simulink library "Garbage"  (R2023a)
settings: Solver=ode45  StartTime=0.0  StopTime=10.0  RelTol=1e-3  EnableMultiTasking=on
undefined symbols (not defined in the file or in its folder): c, ending_column_index, ending_row_index, framebuffer_16x1, framebuffer_2x2, framebuffer_4x4, framebuffer_8x8, HEIGHT, hot, POS_X, POS_Y, starting_column_index, starting_row_index, WIDTH
libraries: Memory_Blocks_Lib x459, simulink x235, simulink_extras x132, dspmtrx3 x116, Bit_Multiplexers_Lib x67, Bit_Demultiplexers_Lib x49, Byte_Multiplexers_Lib x40, Miscellaneous x38, Computer_Library x17, Decoders_Lib x14, commutil2 x12, Arithmetic_Operations_Lib x8
(part 17 of 26)

== system Garbage/Selector_01 ==
  Addr(1B) : Inport
  Bus Selector : BusSelector  OutputSignals=signal1,signal2,signal3,signal4,signal5,signal6,signal7,signal8
  Logical Operator{1..6,26} (7 alike) : Logic  Operator=NOT  Inputs=1  IconShape=distinctive  AllPortsSameDT=off  OutDataTypeStr=boolean
  Logical Operator9 : Logic  Inputs=8  IconShape=distinctive  AllPortsSameDT=off  OutDataTypeStr=boolean
  Select_1(1b) : Outport
  wires:
  Bus Selector/8 -> Logical Operator9/8  "<signal8>"
  Bus Selector/7 -> Logical Operator6/1  "<signal7>"
  Logical Operator6/1 -> Logical Operator9/7
  Bus Selector/6 -> Logical Operator5/1  "<signal6>"
  Logical Operator5/1 -> Logical Operator9/6
  Bus Selector/5 -> Logical Operator4/1  "<signal5>"
  Logical Operator4/1 -> Logical Operator9/5
  Bus Selector/4 -> Logical Operator3/1  "<signal4>"
  Logical Operator3/1 -> Logical Operator9/4
  Bus Selector/3 -> Logical Operator2/1  "<signal3>"
  Logical Operator2/1 -> Logical Operator9/3
  Bus Selector/2 -> Logical Operator1/1  "<signal2>"
  Logical Operator1/1 -> Logical Operator9/2
  Logical Operator9/1 -> Select_1(1b)/1
  Bus Selector/1 -> Logical Operator26/1  "<signal1>"
  Logical Operator26/1 -> Logical Operator9/1
  Addr(1B)/1 -> Bus Selector/1

== system Garbage/Selector_02 ==
  Addr(1B) : Inport
  Bus Selector : BusSelector  OutputSignals=signal1,signal2,signal3,signal4,signal5,signal6,signal7,signal8
  Logical Operator{1..5,7,26} (7 alike) : Logic  Operator=NOT  Inputs=1  IconShape=distinctive  AllPortsSameDT=off  OutDataTypeStr=boolean
  Logical Operator9 : Logic  Inputs=8  IconShape=distinctive  AllPortsSameDT=off  OutDataTypeStr=boolean
  Select_2(1b) : Outport
  wires:
  Bus Selector/8 -> Logical Operator7/1  "<signal8>"
  Logical Operator7/1 -> Logical Operator9/8
  Bus Selector/7 -> Logical Operator9/7  "<signal7>"
  Bus Selector/6 -> Logical Operator5/1  "<signal6>"
  Logical Operator5/1 -> Logical Operator9/6
  Bus Selector/5 -> Logical Operator4/1  "<signal5>"
  Logical Operator4/1 -> Logical Operator9/5
  Bus Selector/4 -> Logical Operator3/1  "<signal4>"
  Logical Operator3/1 -> Logical Operator9/4
  Bus Selector/3 -> Logical Operator2/1  "<signal3>"
  Logical Operator2/1 -> Logical Operator9/3
  Bus Selector/2 -> Logical Operator1/1  "<signal2>"
  Logical Operator1/1 -> Logical Operator9/2
  Logical Operator9/1 -> Select_2(1b)/1
  Bus Selector/1 -> Logical Operator26/1  "<signal1>"
  Logical Operator26/1 -> Logical Operator9/1
  Addr(1B)/1 -> Bus Selector/1

== system Garbage/Selector_03 ==
  Addr(1B) : Inport
  Bus Selector : BusSelector  OutputSignals=signal1,signal2,signal3,signal4,signal5,signal6,signal7,signal8
  Logical Operator{1..5,26} (6 alike) : Logic  Operator=NOT  Inputs=1  IconShape=distinctive  AllPortsSameDT=off  OutDataTypeStr=boolean
  Logical Operator9 : Logic  Inputs=8  IconShape=distinctive  AllPortsSameDT=off  OutDataTypeStr=boolean
  Select_3(1b) : Outport
  wires:
  Bus Selector/8 -> Logical Operator9/8  "<signal8>"
  Bus Selector/7 -> Logical Operator9/7  "<signal7>"
  Bus Selector/6 -> Logical Operator5/1  "<signal6>"
  Logical Operator5/1 -> Logical Operator9/6
  Bus Selector/5 -> Logical Operator4/1  "<signal5>"
  Logical Operator4/1 -> Logical Operator9/5
  Bus Selector/4 -> Logical Operator3/1  "<signal4>"
  Logical Operator3/1 -> Logical Operator9/4
  Bus Selector/3 -> Logical Operator2/1  "<signal3>"
  Logical Operator2/1 -> Logical Operator9/3
  Bus Selector/2 -> Logical Operator1/1  "<signal2>"
  Logical Operator1/1 -> Logical Operator9/2
  Logical Operator9/1 -> Select_3(1b)/1
  Bus Selector/1 -> Logical Operator26/1  "<signal1>"
  Logical Operator26/1 -> Logical Operator9/1
  Addr(1B)/1 -> Bus Selector/1

== system Garbage/Selector_04 ==
  Addr(1B) : Inport
  Bus Selector : BusSelector  OutputSignals=signal1,signal2,signal3,signal4,signal5,signal6,signal7,signal8
  Logical Operator{1..4,6..7,26} (7 alike) : Logic  Operator=NOT  Inputs=1  IconShape=distinctive  AllPortsSameDT=off  OutDataTypeStr=boolean
  Logical Operator9 : Logic  Inputs=8  IconShape=distinctive  AllPortsSameDT=off  OutDataTypeStr=boolean
  Select_4(1b) : Outport
  wires:
  Bus Selector/8 -> Logical Operator7/1  "<signal8>"
  Logical Operator7/1 -> Logical Operator9/8
  Bus Selector/7 -> Logical Operator6/1  "<signal7>"
  Logical Operator6/1 -> Logical Operator9/7
  Bus Selector/6 -> Logical Operator9/6  "<signal6>"
  Bus Selector/5 -> Logical Operator4/1  "<signal5>"
  Logical Operator4/1 -> Logical Operator9/5
  Bus Selector/4 -> Logical Operator3/1  "<signal4>"
  Logical Operator3/1 -> Logical Operator9/4
  Bus Selector/3 -> Logical Operator2/1  "<signal3>"
  Logical Operator2/1 -> Logical Operator9/3
  Bus Selector/2 -> Logical Operator1/1  "<signal2>"
  Logical Operator1/1 -> Logical Operator9/2
  Logical Operator9/1 -> Select_4(1b)/1
  Bus Selector/1 -> Logical Operator26/1  "<signal1>"
  Logical Operator26/1 -> Logical Operator9/1
  Addr(1B)/1 -> Bus Selector/1

== system Garbage/Selector_05 ==
  Addr(1B) : Inport
  Bus Selector : BusSelector  OutputSignals=signal1,signal2,signal3,signal4,signal5,signal6,signal7,signal8
  Logical Operator{1..4,6,26} (6 alike) : Logic  Operator=NOT  Inputs=1  IconShape=distinctive  AllPortsSameDT=off  OutDataTypeStr=boolean
  Logical Operator9 : Logic  Inputs=8  IconShape=distinctive  AllPortsSameDT=off  OutDataTypeStr=boolean
  Select_5(1b) : Outport
  wires:
  Bus Selector/8 -> Logical Operator9/8  "<signal8>"
  Bus Selector/7 -> Logical Operator6/1  "<signal7>"
  Logical Operator6/1 -> Logical Operator9/7
  Bus Selector/6 -> Logical Operator9/6  "<signal6>"
  Bus Selector/5 -> Logical Operator4/1  "<signal5>"
  Logical Operator4/1 -> Logical Operator9/5
  Bus Selector/4 -> Logical Operator3/1  "<signal4>"
  Logical Operator3/1 -> Logical Operator9/4
  Bus Selector/3 -> Logical Operator2/1  "<signal3>"
  Logical Operator2/1 -> Logical Operator9/3
  Bus Selector/2 -> Logical Operator1/1  "<signal2>"
  Logical Operator1/1 -> Logical Operator9/2
  Logical Operator9/1 -> Select_5(1b)/1
  Bus Selector/1 -> Logical Operator26/1  "<signal1>"
  Logical Operator26/1 -> Logical Operator9/1
  Addr(1B)/1 -> Bus Selector/1

== system Garbage/Selector_06 ==
  Addr(1B) : Inport
  Bus Selector : BusSelector  OutputSignals=signal1,signal2,signal3,signal4,signal5,signal6,signal7,signal8
  Logical Operator{1..4,7,26} (6 alike) : Logic  Operator=NOT  Inputs=1  IconShape=distinctive  AllPortsSameDT=off  OutDataTypeStr=boolean
  Logical Operator9 : Logic  Inputs=8  IconShape=distinctive  AllPortsSameDT=off  OutDataTypeStr=boolean
  Select_6(1b) : Outport
  wires:
  Bus Selector/8 -> Logical Operator7/1  "<signal8>"
  Logical Operator7/1 -> Logical Operator9/8
  Bus Selector/7 -> Logical Operator9/7  "<signal7>"
  Bus Selector/6 -> Logical Operator9/6  "<signal6>"
  Bus Selector/5 -> Logical Operator4/1  "<signal5>"
  Logical Operator4/1 -> Logical Operator9/5
  Bus Selector/4 -> Logical Operator3/1  "<signal4>"
  Logical Operator3/1 -> Logical Operator9/4
  Bus Selector/3 -> Logical Operator2/1  "<signal3>"
  Logical Operator2/1 -> Logical Operator9/3
  Bus Selector/2 -> Logical Operator1/1  "<signal2>"
  Logical Operator1/1 -> Logical Operator9/2
  Logical Operator9/1 -> Select_6(1b)/1
  Bus Selector/1 -> Logical Operator26/1  "<signal1>"
  Logical Operator26/1 -> Logical Operator9/1
  Addr(1B)/1 -> Bus Selector/1

== system Garbage/Selector_07 ==
  Addr(1B) : Inport
  Bus Selector : BusSelector  OutputSignals=signal1,signal2,signal3,signal4,signal5,signal6,signal7,signal8
  Logical Operator{1..4,26} (5 alike) : Logic  Operator=NOT  Inputs=1  IconShape=distinctive  AllPortsSameDT=off  OutDataTypeStr=boolean
  Logical Operator9 : Logic  Inputs=8  IconShape=distinctive  AllPortsSameDT=off  OutDataTypeStr=boolean
  Select_7(1b) : Outport
  wires:
  Bus Selector/8 -> Logical Operator9/8  "<signal8>"
  Bus Selector/7 -> Logical Operator9/7  "<signal7>"
  Bus Selector/6 -> Logical Operator9/6  "<signal6>"
  Bus Selector/5 -> Logical Operator4/1  "<signal5>"
  Logical Operator4/1 -> Logical Operator9/5
  Bus Selector/4 -> Logical Operator3/1  "<signal4>"
  Logical Operator3/1 -> Logical Operator9/4
  Bus Selector/3 -> Logical Operator2/1  "<signal3>"
  Logical Operator2/1 -> Logical Operator9/3
  Bus Selector/2 -> Logical Operator1/1  "<signal2>"
  Logical Operator1/1 -> Logical Operator9/2
  Logical Operator9/1 -> Select_7(1b)/1
  Bus Selector/1 -> Logical Operator26/1  "<signal1>"
  Logical Operator26/1 -> Logical Operator9/1
  Addr(1B)/1 -> Bus Selector/1

== system Garbage/Selector_08 ==
  Addr(1B) : Inport
  Bus Selector : BusSelector  OutputSignals=signal1,signal2,signal3,signal4,signal5,signal6,signal7,signal8
  Logical Operator{1..3,5..7,26} (7 alike) : Logic  Operator=NOT  Inputs=1  IconShape=distinctive  AllPortsSameDT=off  OutDataTypeStr=boolean
  Logical Operator9 : Logic  Inputs=8  IconShape=distinctive  AllPortsSameDT=off  OutDataTypeStr=boolean
  Select_8(1b) : Outport
  wires:
  Bus Selector/8 -> Logical Operator7/1  "<signal8>"
  Logical Operator7/1 -> Logical Operator9/8
  Bus Selector/7 -> Logical Operator6/1  "<signal7>"
  Logical Operator6/1 -> Logical Operator9/7
  Bus Selector/6 -> Logical Operator5/1  "<signal6>"
  Logical Operator5/1 -> Logical Operator9/6
  Bus Selector/5 -> Logical Operator9/5  "<signal5>"
  Bus Selector/4 -> Logical Operator3/1  "<signal4>"
  Logical Operator3/1 -> Logical Operator9/4
  Bus Selector/3 -> Logical Operator2/1  "<signal3>"
  Logical Operator2/1 -> Logical Operator9/3
  Bus Selector/2 -> Logical Operator1/1  "<signal2>"
  Logical Operator1/1 -> Logical Operator9/2
  Logical Operator9/1 -> Select_8(1b)/1
  Bus Selector/1 -> Logical Operator26/1  "<signal1>"
  Logical Operator26/1 -> Logical Operator9/1
  Addr(1B)/1 -> Bus Selector/1

== system Garbage/Selector_09 ==
  Addr(1B) : Inport
  Bus Selector : BusSelector  OutputSignals=signal1,signal2,signal3,signal4,signal5,signal6,signal7,signal8
  Logical Operator{1..3,5..6,26} (6 alike) : Logic  Operator=NOT  Inputs=1  IconShape=distinctive  AllPortsSameDT=off  OutDataTypeStr=boolean
  Logical Operator9 : Logic  Inputs=8  IconShape=distinctive  AllPortsSameDT=off  OutDataTypeStr=boolean
  Select_9(1b) : Outport
  wires:
  Bus Selector/8 -> Logical Operator9/8  "<signal8>"
  Bus Selector/7 -> Logical Operator6/1  "<signal7>"
  Logical Operator6/1 -> Logical Operator9/7
  Bus Selector/6 -> Logical Operator5/1  "<signal6>"
  Logical Operator5/1 -> Logical Operator9/6
  Bus Selector/5 -> Logical Operator9/5  "<signal5>"
  Bus Selector/4 -> Logical Operator3/1  "<signal4>"
  Logical Operator3/1 -> Logical Operator9/4
  Bus Selector/3 -> Logical Operator2/1  "<signal3>"
  Logical Operator2/1 -> Logical Operator9/3
  Bus Selector/2 -> Logical Operator1/1  "<signal2>"
  Logical Operator1/1 -> Logical Operator9/2
  Logical Operator9/1 -> Select_9(1b)/1
  Bus Selector/1 -> Logical Operator26/1  "<signal1>"
  Logical Operator26/1 -> Logical Operator9/1
  Addr(1B)/1 -> Bus Selector/1

== system Garbage/Selector_10 ==
  Addr(1B) : Inport
  Bus Selector : BusSelector  OutputSignals=signal1,signal2,signal3,signal4,signal5,signal6,signal7,signal8
  Logical Operator{1..3,5,7,26} (6 alike) : Logic  Operator=NOT  Inputs=1  IconShape=distinctive  AllPortsSameDT=off  OutDataTypeStr=boolean
  Logical Operator9 : Logic  Inputs=8  IconShape=distinctive  AllPortsSameDT=off  OutDataTypeStr=boolean
  Select_10(1b) : Outport
  wires:
  Bus Selector/8 -> Logical Operator7/1  "<signal8>"
  Logical Operator7/1 -> Logical Operator9/8
  Bus Selector/7 -> Logical Operator9/7  "<signal7>"
  Bus Selector/6 -> Logical Operator5/1  "<signal6>"
  Logical Operator5/1 -> Logical Operator9/6
  Bus Selector/5 -> Logical Operator9/5  "<signal5>"
  Bus Selector/4 -> Logical Operator3/1  "<signal4>"
  Logical Operator3/1 -> Logical Operator9/4
  Bus Selector/3 -> Logical Operator2/1  "<signal3>"
  Logical Operator2/1 -> Logical Operator9/3
  Bus Selector/2 -> Logical Operator1/1  "<signal2>"
  Logical Operator1/1 -> Logical Operator9/2
  Logical Operator9/1 -> Select_10(1b)/1
  Bus Selector/1 -> Logical Operator26/1  "<signal1>"
  Logical Operator26/1 -> Logical Operator9/1
  Addr(1B)/1 -> Bus Selector/1

== system Garbage/Selector_11 ==
  Addr(1B) : Inport
  Bus Selector : BusSelector  OutputSignals=signal1,signal2,signal3,signal4,signal5,signal6,signal7,signal8
  Logical Operator{1..3,5,26} (5 alike) : Logic  Operator=NOT  Inputs=1  IconShape=distinctive  AllPortsSameDT=off  OutDataTypeStr=boolean
  Logical Operator9 : Logic  Inputs=8  IconShape=distinctive  AllPortsSameDT=off  OutDataTypeStr=boolean
  Select_11(1b) : Outport
  wires:
  Bus Selector/8 -> Logical Operator9/8  "<signal8>"
  Bus Selector/7 -> Logical Operator9/7  "<signal7>"
  Bus Selector/6 -> Logical Operator5/1  "<signal6>"
  Logical Operator5/1 -> Logical Operator9/6
  Bus Selector/5 -> Logical Operator9/5  "<signal5>"
  Bus Selector/4 -> Logical Operator3/1  "<signal4>"
  Logical Operator3/1 -> Logical Operator9/4
  Bus Selector/3 -> Logical Operator2/1  "<signal3>"
  Logical Operator2/1 -> Logical Operator9/3
  Bus Selector/2 -> Logical Operator1/1  "<signal2>"
  Logical Operator1/1 -> Logical Operator9/2
  Logical Operator9/1 -> Select_11(1b)/1
  Bus Selector/1 -> Logical Operator26/1  "<signal1>"
  Logical Operator26/1 -> Logical Operator9/1
  Addr(1B)/1 -> Bus Selector/1

== system Garbage/Selector_12 ==
  Addr(1B) : Inport
  Bus Selector : BusSelector  OutputSignals=signal1,signal2,signal3,signal4,signal5,signal6,signal7,signal8
  Logical Operator{1..3,6..7,26} (6 alike) : Logic  Operator=NOT  Inputs=1  IconShape=distinctive  AllPortsSameDT=off  OutDataTypeStr=boolean
  Logical Operator9 : Logic  Inputs=8  IconShape=distinctive  AllPortsSameDT=off  OutDataTypeStr=boolean
  Select_12(1b) : Outport
  wires:
  Bus Selector/8 -> Logical Operator7/1  "<signal8>"
  Logical Operator7/1 -> Logical Operator9/8
  Bus Selector/7 -> Logical Operator6/1  "<signal7>"
  Logical Operator6/1 -> Logical Operator9/7
  Bus Selector/6 -> Logical Operator9/6  "<signal6>"
  Bus Selector/5 -> Logical Operator9/5  "<signal5>"
  Bus Selector/4 -> Logical Operator3/1  "<signal4>"
  Logical Operator3/1 -> Logical Operator9/4
  Bus Selector/3 -> Logical Operator2/1  "<signal3>"
  Logical Operator2/1 -> Logical Operator9/3
  Bus Selector/2 -> Logical Operator1/1  "<signal2>"
  Logical Operator1/1 -> Logical Operator9/2
  Logical Operator9/1 -> Select_12(1b)/1
  Bus Selector/1 -> Logical Operator26/1  "<signal1>"
  Logical Operator26/1 -> Logical Operator9/1
  Addr(1B)/1 -> Bus Selector/1

== system Garbage/Selector_13 ==
  Addr(1B) : Inport
  Bus Selector : BusSelector  OutputSignals=signal1,signal2,signal3,signal4,signal5,signal6,signal7,signal8
  Logical Operator{1..3,6,26} (5 alike) : Logic  Operator=NOT  Inputs=1  IconShape=distinctive  AllPortsSameDT=off  OutDataTypeStr=boolean
  Logical Operator9 : Logic  Inputs=8  IconShape=distinctive  AllPortsSameDT=off  OutDataTypeStr=boolean
  Select_13(1b) : Outport
  wires:
  Bus Selector/8 -> Logical Operator9/8  "<signal8>"
  Bus Selector/7 -> Logical Operator6/1  "<signal7>"
  Logical Operator6/1 -> Logical Operator9/7
  Bus Selector/6 -> Logical Operator9/6  "<signal6>"
  Bus Selector/5 -> Logical Operator9/5  "<signal5>"
  Bus Selector/4 -> Logical Operator3/1  "<signal4>"
  Logical Operator3/1 -> Logical Operator9/4
  Bus Selector/3 -> Logical Operator2/1  "<signal3>"
  Logical Operator2/1 -> Logical Operator9/3
  Bus Selector/2 -> Logical Operator1/1  "<signal2>"
  Logical Operator1/1 -> Logical Operator9/2
  Logical Operator9/1 -> Select_13(1b)/1
  Bus Selector/1 -> Logical Operator26/1  "<signal1>"
  Logical Operator26/1 -> Logical Operator9/1
  Addr(1B)/1 -> Bus Selector/1

== system Garbage/Selector_14 ==
  Addr(1B) : Inport
  Bus Selector : BusSelector  OutputSignals=signal1,signal2,signal3,signal4,signal5,signal6,signal7,signal8
  Logical Operator{1..3,7,26} (5 alike) : Logic  Operator=NOT  Inputs=1  IconShape=distinctive  AllPortsSameDT=off  OutDataTypeStr=boolean
  Logical Operator9 : Logic  Inputs=8  IconShape=distinctive  AllPortsSameDT=off  OutDataTypeStr=boolean
  Select_14(1b) : Outport
  wires:
  Bus Selector/8 -> Logical Operator7/1  "<signal8>"
  Logical Operator7/1 -> Logical Operator9/8
  Bus Selector/7 -> Logical Operator9/7  "<signal7>"
  Bus Selector/6 -> Logical Operator9/6  "<signal6>"
  Bus Selector/5 -> Logical Operator9/5  "<signal5>"
  Bus Selector/4 -> Logical Operator3/1  "<signal4>"
  Logical Operator3/1 -> Logical Operator9/4
  Bus Selector/3 -> Logical Operator2/1  "<signal3>"
  Logical Operator2/1 -> Logical Operator9/3
  Bus Selector/2 -> Logical Operator1/1  "<signal2>"
  Logical Operator1/1 -> Logical Operator9/2
  Logical Operator9/1 -> Select_14(1b)/1
  Bus Selector/1 -> Logical Operator26/1  "<signal1>"
  Logical Operator26/1 -> Logical Operator9/1
  Addr(1B)/1 -> Bus Selector/1

== system Garbage/Selector_15 ==
  Addr(1B) : Inport
  Bus Selector : BusSelector  OutputSignals=signal1,signal2,signal3,signal4,signal5,signal6,signal7,signal8
  Logical Operator{1..3,26} (4 alike) : Logic  Operator=NOT  Inputs=1  IconShape=distinctive  AllPortsSameDT=off  OutDataTypeStr=boolean
  Logical Operator9 : Logic  Inputs=8  IconShape=distinctive  AllPortsSameDT=off  OutDataTypeStr=boolean
  Select_15(1b) : Outport
  wires:
  Bus Selector/8 -> Logical Operator9/8  "<signal8>"
  Bus Selector/7 -> Logical Operator9/7  "<signal7>"
  Bus Selector/6 -> Logical Operator9/6  "<signal6>"
  Bus Selector/5 -> Logical Operator9/5  "<signal5>"
  Bus Selector/4 -> Logical Operator3/1  "<signal4>"
  Logical Operator3/1 -> Logical Operator9/4
  Bus Selector/3 -> Logical Operator2/1  "<signal3>"
  Logical Operator2/1 -> Logical Operator9/3
  Bus Selector/2 -> Logical Operator1/1  "<signal2>"
  Logical Operator1/1 -> Logical Operator9/2
  Logical Operator9/1 -> Select_15(1b)/1
  Bus Selector/1 -> Logical Operator26/1  "<signal1>"
  Logical Operator26/1 -> Logical Operator9/1
  Addr(1B)/1 -> Bus Selector/1

== system Garbage/Selector_16 ==
  Addr(1B) : Inport
  Bus Selector : BusSelector  OutputSignals=signal1,signal2,signal3,signal4,signal5,signal6,signal7,signal8
  Logical Operator{1..2,4..7,26} (7 alike) : Logic  Operator=NOT  Inputs=1  IconShape=distinctive  AllPortsSameDT=off  OutDataTypeStr=boolean
  Logical Operator9 : Logic  Inputs=8  IconShape=distinctive  AllPortsSameDT=off  OutDataTypeStr=boolean
  Select_16(1b) : Outport
  wires:
  Bus Selector/8 -> Logical Operator7/1  "<signal8>"
  Logical Operator7/1 -> Logical Operator9/8
  Bus Selector/7 -> Logical Operator6/1  "<signal7>"
  Logical Operator6/1 -> Logical Operator9/7
  Bus Selector/6 -> Logical Operator5/1  "<signal6>"
  Logical Operator5/1 -> Logical Operator9/6
  Bus Selector/5 -> Logical Operator4/1  "<signal5>"
  Logical Operator4/1 -> Logical Operator9/5
  Bus Selector/4 -> Logical Operator9/4  "<signal4>"
  Bus Selector/3 -> Logical Operator2/1  "<signal3>"
  Logical Operator2/1 -> Logical Operator9/3
  Bus Selector/2 -> Logical Operator1/1  "<signal2>"
  Logical Operator1/1 -> Logical Operator9/2
  Logical Operator9/1 -> Select_16(1b)/1
  Bus Selector/1 -> Logical Operator26/1  "<signal1>"
  Logical Operator26/1 -> Logical Operator9/1
  Addr(1B)/1 -> Bus Selector/1

== system Garbage/Selector_17 ==
  Addr(1B) : Inport
  Bus Selector : BusSelector  OutputSignals=signal1,signal2,signal3,signal4,signal5,signal6,signal7,signal8
  Logical Operator{1..2,4..6,26} (6 alike) : Logic  Operator=NOT  Inputs=1  IconShape=distinctive  AllPortsSameDT=off  OutDataTypeStr=boolean
  Logical Operator9 : Logic  Inputs=8  IconShape=distinctive  AllPortsSameDT=off  OutDataTypeStr=boolean
  Select_17(1b) : Outport
  wires:
  Bus Selector/8 -> Logical Operator9/8  "<signal8>"
  Bus Selector/7 -> Logical Operator6/1  "<signal7>"
  Logical Operator6/1 -> Logical Operator9/7
  Bus Selector/6 -> Logical Operator5/1  "<signal6>"
  Logical Operator5/1 -> Logical Operator9/6
  Bus Selector/5 -> Logical Operator4/1  "<signal5>"
  Logical Operator4/1 -> Logical Operator9/5
  Bus Selector/4 -> Logical Operator9/4  "<signal4>"
  Bus Selector/3 -> Logical Operator2/1  "<signal3>"
  Logical Operator2/1 -> Logical Operator9/3
  Bus Selector/2 -> Logical Operator1/1  "<signal2>"
  Logical Operator1/1 -> Logical Operator9/2
  Logical Operator9/1 -> Select_17(1b)/1
  Bus Selector/1 -> Logical Operator26/1  "<signal1>"
  Logical Operator26/1 -> Logical Operator9/1
  Addr(1B)/1 -> Bus Selector/1

== system Garbage/Selector_18 ==
  Addr(1B) : Inport
  Bus Selector : BusSelector  OutputSignals=signal1,signal2,signal3,signal4,signal5,signal6,signal7,signal8
  Logical Operator{1..2,4..5,7,26} (6 alike) : Logic  Operator=NOT  Inputs=1  IconShape=distinctive  AllPortsSameDT=off  OutDataTypeStr=boolean
  Logical Operator9 : Logic  Inputs=8  IconShape=distinctive  AllPortsSameDT=off  OutDataTypeStr=boolean
  Select_18(1b) : Outport
  wires:
  Bus Selector/8 -> Logical Operator7/1  "<signal8>"
  Logical Operator7/1 -> Logical Operator9/8
  Bus Selector/7 -> Logical Operator9/7  "<signal7>"
  Bus Selector/6 -> Logical Operator5/1  "<signal6>"
  Logical Operator5/1 -> Logical Operator9/6
  Bus Selector/5 -> Logical Operator4/1  "<signal5>"
  Logical Operator4/1 -> Logical Operator9/5
  Bus Selector/4 -> Logical Operator9/4  "<signal4>"
  Bus Selector/3 -> Logical Operator2/1  "<signal3>"
  Logical Operator2/1 -> Logical Operator9/3
  Bus Selector/2 -> Logical Operator1/1  "<signal2>"
  Logical Operator1/1 -> Logical Operator9/2
  Logical Operator9/1 -> Select_18(1b)/1
  Bus Selector/1 -> Logical Operator26/1  "<signal1>"
  Logical Operator26/1 -> Logical Operator9/1
  Addr(1B)/1 -> Bus Selector/1

== system Garbage/Selector_19 ==
  Addr(1B) : Inport
  Bus Selector : BusSelector  OutputSignals=signal1,signal2,signal3,signal4,signal5,signal6,signal7,signal8
  Logical Operator{1..2,4..5,26} (5 alike) : Logic  Operator=NOT  Inputs=1  IconShape=distinctive  AllPortsSameDT=off  OutDataTypeStr=boolean
  Logical Operator9 : Logic  Inputs=8  IconShape=distinctive  AllPortsSameDT=off  OutDataTypeStr=boolean
  Select_19(1b) : Outport
  wires:
  Bus Selector/8 -> Logical Operator9/8  "<signal8>"
  Bus Selector/7 -> Logical Operator9/7  "<signal7>"
  Bus Selector/6 -> Logical Operator5/1  "<signal6>"
  Logical Operator5/1 -> Logical Operator9/6
  Bus Selector/5 -> Logical Operator4/1  "<signal5>"
  Logical Operator4/1 -> Logical Operator9/5
  Bus Selector/4 -> Logical Operator9/4  "<signal4>"
  Bus Selector/3 -> Logical Operator2/1  "<signal3>"
  Logical Operator2/1 -> Logical Operator9/3
  Bus Selector/2 -> Logical Operator1/1  "<signal2>"
  Logical Operator1/1 -> Logical Operator9/2
  Logical Operator9/1 -> Select_19(1b)/1
  Bus Selector/1 -> Logical Operator26/1  "<signal1>"
  Logical Operator26/1 -> Logical Operator9/1
  Addr(1B)/1 -> Bus Selector/1

== system Garbage/Selector_20 ==
  Addr(1B) : Inport
  Bus Selector : BusSelector  OutputSignals=signal1,signal2,signal3,signal4,signal5,signal6,signal7,signal8
  Logical Operator{1..2,4,6..7,26} (6 alike) : Logic  Operator=NOT  Inputs=1  IconShape=distinctive  AllPortsSameDT=off  OutDataTypeStr=boolean
  Logical Operator9 : Logic  Inputs=8  IconShape=distinctive  AllPortsSameDT=off  OutDataTypeStr=boolean
  Select_20(1b) : Outport
  wires:
  Bus Selector/8 -> Logical Operator7/1  "<signal8>"
  Logical Operator7/1 -> Logical Operator9/8
  Bus Selector/7 -> Logical Operator6/1  "<signal7>"
  Logical Operator6/1 -> Logical Operator9/7
  Bus Selector/6 -> Logical Operator9/6  "<signal6>"
  Bus Selector/5 -> Logical Operator4/1  "<signal5>"
  Logical Operator4/1 -> Logical Operator9/5
  Bus Selector/4 -> Logical Operator9/4  "<signal4>"
  Bus Selector/3 -> Logical Operator2/1  "<signal3>"
  Logical Operator2/1 -> Logical Operator9/3
  Bus Selector/2 -> Logical Operator1/1  "<signal2>"
  Logical Operator1/1 -> Logical Operator9/2
  Logical Operator9/1 -> Select_20(1b)/1
  Bus Selector/1 -> Logical Operator26/1  "<signal1>"
  Logical Operator26/1 -> Logical Operator9/1
  Addr(1B)/1 -> Bus Selector/1

== system Garbage/Selector_21 ==
  Addr(1B) : Inport
  Bus Selector : BusSelector  OutputSignals=signal1,signal2,signal3,signal4,signal5,signal6,signal7,signal8
  Logical Operator{1..2,4,6,26} (5 alike) : Logic  Operator=NOT  Inputs=1  IconShape=distinctive  AllPortsSameDT=off  OutDataTypeStr=boolean
  Logical Operator9 : Logic  Inputs=8  IconShape=distinctive  AllPortsSameDT=off  OutDataTypeStr=boolean
  Select_21(1b) : Outport
  wires:
  Bus Selector/8 -> Logical Operator9/8  "<signal8>"
  Bus Selector/7 -> Logical Operator6/1  "<signal7>"
  Logical Operator6/1 -> Logical Operator9/7
  Bus Selector/6 -> Logical Operator9/6  "<signal6>"
  Bus Selector/5 -> Logical Operator4/1  "<signal5>"
  Logical Operator4/1 -> Logical Operator9/5
  Bus Selector/4 -> Logical Operator9/4  "<signal4>"
  Bus Selector/3 -> Logical Operator2/1  "<signal3>"
  Logical Operator2/1 -> Logical Operator9/3
  Bus Selector/2 -> Logical Operator1/1  "<signal2>"
  Logical Operator1/1 -> Logical Operator9/2
  Logical Operator9/1 -> Select_21(1b)/1
  Bus Selector/1 -> Logical Operator26/1  "<signal1>"
  Logical Operator26/1 -> Logical Operator9/1
  Addr(1B)/1 -> Bus Selector/1

== system Garbage/Selector_22 ==
  Addr(1B) : Inport
  Bus Selector : BusSelector  OutputSignals=signal1,signal2,signal3,signal4,signal5,signal6,signal7,signal8
  Logical Operator{1..2,4,7,26} (5 alike) : Logic  Operator=NOT  Inputs=1  IconShape=distinctive  AllPortsSameDT=off  OutDataTypeStr=boolean
  Logical Operator9 : Logic  Inputs=8  IconShape=distinctive  AllPortsSameDT=off  OutDataTypeStr=boolean
  Select_22(1b) : Outport
  wires:
  Bus Selector/8 -> Logical Operator7/1  "<signal8>"
  Logical Operator7/1 -> Logical Operator9/8
  Bus Selector/7 -> Logical Operator9/7  "<signal7>"
  Bus Selector/6 -> Logical Operator9/6  "<signal6>"
  Bus Selector/5 -> Logical Operator4/1  "<signal5>"
  Logical Operator4/1 -> Logical Operator9/5
  Bus Selector/4 -> Logical Operator9/4  "<signal4>"
  Bus Selector/3 -> Logical Operator2/1  "<signal3>"
  Logical Operator2/1 -> Logical Operator9/3
  Bus Selector/2 -> Logical Operator1/1  "<signal2>"
  Logical Operator1/1 -> Logical Operator9/2
  Logical Operator9/1 -> Select_22(1b)/1
  Bus Selector/1 -> Logical Operator26/1  "<signal1>"
  Logical Operator26/1 -> Logical Operator9/1
  Addr(1B)/1 -> Bus Selector/1

== system Garbage/Selector_23 ==
  Addr(1B) : Inport
  Bus Selector : BusSelector  OutputSignals=signal1,signal2,signal3,signal4,signal5,signal6,signal7,signal8
  Logical Operator{1..2,4,26} (4 alike) : Logic  Operator=NOT  Inputs=1  IconShape=distinctive  AllPortsSameDT=off  OutDataTypeStr=boolean
  Logical Operator9 : Logic  Inputs=8  IconShape=distinctive  AllPortsSameDT=off  OutDataTypeStr=boolean
  Select_23(1b) : Outport
  wires:
  Bus Selector/8 -> Logical Operator9/8  "<signal8>"
  Bus Selector/7 -> Logical Operator9/7  "<signal7>"
  Bus Selector/6 -> Logical Operator9/6  "<signal6>"
  Bus Selector/5 -> Logical Operator4/1  "<signal5>"
  Logical Operator4/1 -> Logical Operator9/5
  Bus Selector/4 -> Logical Operator9/4  "<signal4>"
  Bus Selector/3 -> Logical Operator2/1  "<signal3>"
  Logical Operator2/1 -> Logical Operator9/3
  Bus Selector/2 -> Logical Operator1/1  "<signal2>"
  Logical Operator1/1 -> Logical Operator9/2
  Logical Operator9/1 -> Select_23(1b)/1
  Bus Selector/1 -> Logical Operator26/1  "<signal1>"
  Logical Operator26/1 -> Logical Operator9/1
  Addr(1B)/1 -> Bus Selector/1

== system Garbage/Selector_24 ==
  Addr(1B) : Inport
  Bus Selector : BusSelector  OutputSignals=signal1,signal2,signal3,signal4,signal5,signal6,signal7,signal8
  Logical Operator{1..2,5..7,26} (6 alike) : Logic  Operator=NOT  Inputs=1  IconShape=distinctive  AllPortsSameDT=off  OutDataTypeStr=boolean
  Logical Operator9 : Logic  Inputs=8  IconShape=distinctive  AllPortsSameDT=off  OutDataTypeStr=boolean
  Select_24(1b) : Outport
  wires:
  Bus Selector/8 -> Logical Operator7/1  "<signal8>"
  Logical Operator7/1 -> Logical Operator9/8
  Bus Selector/7 -> Logical Operator6/1  "<signal7>"
  Logical Operator6/1 -> Logical Operator9/7
  Bus Selector/6 -> Logical Operator5/1  "<signal6>"
  Logical Operator5/1 -> Logical Operator9/6
  Bus Selector/5 -> Logical Operator9/5  "<signal5>"
  Bus Selector/4 -> Logical Operator9/4  "<signal4>"
  Bus Selector/3 -> Logical Operator2/1  "<signal3>"
  Logical Operator2/1 -> Logical Operator9/3
  Bus Selector/2 -> Logical Operator1/1  "<signal2>"
  Logical Operator1/1 -> Logical Operator9/2
  Logical Operator9/1 -> Select_24(1b)/1
  Bus Selector/1 -> Logical Operator26/1  "<signal1>"
  Logical Operator26/1 -> Logical Operator9/1
  Addr(1B)/1 -> Bus Selector/1

== system Garbage/Selector_25 ==
  Addr(1B) : Inport
  Bus Selector : BusSelector  OutputSignals=signal1,signal2,signal3,signal4,signal5,signal6,signal7,signal8
  Logical Operator{1..2,5..6,26} (5 alike) : Logic  Operator=NOT  Inputs=1  IconShape=distinctive  AllPortsSameDT=off  OutDataTypeStr=boolean
  Logical Operator9 : Logic  Inputs=8  IconShape=distinctive  AllPortsSameDT=off  OutDataTypeStr=boolean
  Select_25(1b) : Outport
  wires:
  Bus Selector/8 -> Logical Operator9/8  "<signal8>"
  Bus Selector/7 -> Logical Operator6/1  "<signal7>"
  Logical Operator6/1 -> Logical Operator9/7
  Bus Selector/6 -> Logical Operator5/1  "<signal6>"
  Logical Operator5/1 -> Logical Operator9/6
  Bus Selector/5 -> Logical Operator9/5  "<signal5>"
  Bus Selector/4 -> Logical Operator9/4  "<signal4>"
  Bus Selector/3 -> Logical Operator2/1  "<signal3>"
  Logical Operator2/1 -> Logical Operator9/3
  Bus Selector/2 -> Logical Operator1/1  "<signal2>"
  Logical Operator1/1 -> Logical Operator9/2
  Logical Operator9/1 -> Select_25(1b)/1
  Bus Selector/1 -> Logical Operator26/1  "<signal1>"
  Logical Operator26/1 -> Logical Operator9/1
  Addr(1B)/1 -> Bus Selector/1

== system Garbage/Selector_26 ==
  Addr(1B) : Inport
  Bus Selector : BusSelector  OutputSignals=signal1,signal2,signal3,signal4,signal5,signal6,signal7,signal8
  Logical Operator{1..2,5,7,26} (5 alike) : Logic  Operator=NOT  Inputs=1  IconShape=distinctive  AllPortsSameDT=off  OutDataTypeStr=boolean
  Logical Operator9 : Logic  Inputs=8  IconShape=distinctive  AllPortsSameDT=off  OutDataTypeStr=boolean
  Select_26(1b) : Outport
  wires:
  Bus Selector/8 -> Logical Operator7/1  "<signal8>"
  Logical Operator7/1 -> Logical Operator9/8
  Bus Selector/7 -> Logical Operator9/7  "<signal7>"
  Bus Selector/6 -> Logical Operator5/1  "<signal6>"
  Logical Operator5/1 -> Logical Operator9/6
  Bus Selector/5 -> Logical Operator9/5  "<signal5>"
  Bus Selector/4 -> Logical Operator9/4  "<signal4>"
  Bus Selector/3 -> Logical Operator2/1  "<signal3>"
  Logical Operator2/1 -> Logical Operator9/3
  Bus Selector/2 -> Logical Operator1/1  "<signal2>"
  Logical Operator1/1 -> Logical Operator9/2
  Logical Operator9/1 -> Select_26(1b)/1
  Bus Selector/1 -> Logical Operator26/1  "<signal1>"
  Logical Operator26/1 -> Logical Operator9/1
  Addr(1B)/1 -> Bus Selector/1

== system Garbage/Selector_27 ==
  Addr(1B) : Inport
  Bus Selector : BusSelector  OutputSignals=signal1,signal2,signal3,signal4,signal5,signal6,signal7,signal8
  Logical Operator{1..2,5,26} (4 alike) : Logic  Operator=NOT  Inputs=1  IconShape=distinctive  AllPortsSameDT=off  OutDataTypeStr=boolean
  Logical Operator9 : Logic  Inputs=8  IconShape=distinctive  AllPortsSameDT=off  OutDataTypeStr=boolean
  Select_27(1b) : Outport
  wires:
  Bus Selector/8 -> Logical Operator9/8  "<signal8>"
  Bus Selector/7 -> Logical Operator9/7  "<signal7>"
  Bus Selector/6 -> Logical Operator5/1  "<signal6>"
  Logical Operator5/1 -> Logical Operator9/6
  Bus Selector/5 -> Logical Operator9/5  "<signal5>"
  Bus Selector/4 -> Logical Operator9/4  "<signal4>"
  Bus Selector/3 -> Logical Operator2/1  "<signal3>"
  Logical Operator2/1 -> Logical Operator9/3
  Bus Selector/2 -> Logical Operator1/1  "<signal2>"
  Logical Operator1/1 -> Logical Operator9/2
  Logical Operator9/1 -> Select_27(1b)/1
  Bus Selector/1 -> Logical Operator26/1  "<signal1>"
  Logical Operator26/1 -> Logical Operator9/1
  Addr(1B)/1 -> Bus Selector/1

== system Garbage/Selector_28 ==
  Addr(1B) : Inport
  Bus Selector : BusSelector  OutputSignals=signal1,signal2,signal3,signal4,signal5,signal6,signal7,signal8
  Logical Operator{1..2,6..7,26} (5 alike) : Logic  Operator=NOT  Inputs=1  IconShape=distinctive  AllPortsSameDT=off  OutDataTypeStr=boolean
  Logical Operator9 : Logic  Inputs=8  IconShape=distinctive  AllPortsSameDT=off  OutDataTypeStr=boolean
  Select_28(1b) : Outport
  wires:
  Bus Selector/8 -> Logical Operator7/1  "<signal8>"
  Logical Operator7/1 -> Logical Operator9/8
  Bus Selector/7 -> Logical Operator6/1  "<signal7>"
  Logical Operator6/1 -> Logical Operator9/7
  Bus Selector/6 -> Logical Operator9/6  "<signal6>"
  Bus Selector/5 -> Logical Operator9/5  "<signal5>"
  Bus Selector/4 -> Logical Operator9/4  "<signal4>"
  Bus Selector/3 -> Logical Operator2/1  "<signal3>"
  Logical Operator2/1 -> Logical Operator9/3
  Bus Selector/2 -> Logical Operator1/1  "<signal2>"
  Logical Operator1/1 -> Logical Operator9/2
  Logical Operator9/1 -> Select_28(1b)/1
  Bus Selector/1 -> Logical Operator26/1  "<signal1>"
  Logical Operator26/1 -> Logical Operator9/1
  Addr(1B)/1 -> Bus Selector/1

== system Garbage/Selector_29 ==
  Addr(1B) : Inport
  Bus Selector : BusSelector  OutputSignals=signal1,signal2,signal3,signal4,signal5,signal6,signal7,signal8
  Logical Operator{1..2,6,26} (4 alike) : Logic  Operator=NOT  Inputs=1  IconShape=distinctive  AllPortsSameDT=off  OutDataTypeStr=boolean
  Logical Operator9 : Logic  Inputs=8  IconShape=distinctive  AllPortsSameDT=off  OutDataTypeStr=boolean
  Select_29(1b) : Outport
  wires:
  Bus Selector/8 -> Logical Operator9/8  "<signal8>"
  Bus Selector/7 -> Logical Operator6/1  "<signal7>"
  Logical Operator6/1 -> Logical Operator9/7
  Bus Selector/6 -> Logical Operator9/6  "<signal6>"
  Bus Selector/5 -> Logical Operator9/5  "<signal5>"
  Bus Selector/4 -> Logical Operator9/4  "<signal4>"
  Bus Selector/3 -> Logical Operator2/1  "<signal3>"
  Logical Operator2/1 -> Logical Operator9/3
  Bus Selector/2 -> Logical Operator1/1  "<signal2>"
  Logical Operator1/1 -> Logical Operator9/2
  Logical Operator9/1 -> Select_29(1b)/1
  Bus Selector/1 -> Logical Operator26/1  "<signal1>"
  Logical Operator26/1 -> Logical Operator9/1
  Addr(1B)/1 -> Bus Selector/1

== system Garbage/Selector_30 ==
  Addr(1B) : Inport
  Bus Selector : BusSelector  OutputSignals=signal1,signal2,signal3,signal4,signal5,signal6,signal7,signal8
  Logical Operator{1..2,7,26} (4 alike) : Logic  Operator=NOT  Inputs=1  IconShape=distinctive  AllPortsSameDT=off  OutDataTypeStr=boolean
  Logical Operator9 : Logic  Inputs=8  IconShape=distinctive  AllPortsSameDT=off  OutDataTypeStr=boolean
  Select_30(1b) : Outport
  wires:
  Bus Selector/8 -> Logical Operator7/1  "<signal8>"
  Logical Operator7/1 -> Logical Operator9/8
  Bus Selector/7 -> Logical Operator9/7  "<signal7>"
  Bus Selector/6 -> Logical Operator9/6  "<signal6>"
  Bus Selector/5 -> Logical Operator9/5  "<signal5>"
  Bus Selector/4 -> Logical Operator9/4  "<signal4>"
  Bus Selector/3 -> Logical Operator2/1  "<signal3>"
  Logical Operator2/1 -> Logical Operator9/3
  Bus Selector/2 -> Logical Operator1/1  "<signal2>"
  Logical Operator1/1 -> Logical Operator9/2
  Logical Operator9/1 -> Select_30(1b)/1
  Bus Selector/1 -> Logical Operator26/1  "<signal1>"
  Logical Operator26/1 -> Logical Operator9/1
  Addr(1B)/1 -> Bus Selector/1

== system Garbage/Selector_31 ==
  Addr(1B) : Inport
  Bus Selector : BusSelector  OutputSignals=signal1,signal2,signal3,signal4,signal5,signal6,signal7,signal8
  Logical Operator1 : Logic  Operator=NOT  Inputs=1  IconShape=distinctive  AllPortsSameDT=off  OutDataTypeStr=boolean
  Logical Operator2 : Logic  Operator=NOT  Inputs=1  IconShape=distinctive  AllPortsSameDT=off  OutDataTypeStr=boolean
  Logical Operator26 : Logic  Operator=NOT  Inputs=1  IconShape=distinctive  AllPortsSameDT=off  OutDataTypeStr=boolean
  Logical Operator9 : Logic  Inputs=8  IconShape=distinctive  AllPortsSameDT=off  OutDataTypeStr=boolean
  Select_31(1b) : Outport
  wires:
  Bus Selector/8 -> Logical Operator9/8  "<signal8>"
  Bus Selector/7 -> Logical Operator9/7  "<signal7>"
  Bus Selector/6 -> Logical Operator9/6  "<signal6>"
  Bus Selector/5 -> Logical Operator9/5  "<signal5>"
  Bus Selector/4 -> Logical Operator9/4  "<signal4>"
  Bus Selector/3 -> Logical Operator2/1  "<signal3>"
  Logical Operator2/1 -> Logical Operator9/3
  Bus Selector/2 -> Logical Operator1/1  "<signal2>"
  Logical Operator1/1 -> Logical Operator9/2
  Logical Operator9/1 -> Select_31(1b)/1
  Bus Selector/1 -> Logical Operator26/1  "<signal1>"
  Logical Operator26/1 -> Logical Operator9/1
  Addr(1B)/1 -> Bus Selector/1
